simulink model "snap2_f_200msps_64i_4096c_for_8_11_2124fpg"  (R2019a)
settings: Solver=VariableStepDiscrete  StartTime=0.0  StopTime=10.0  RelTol=1e-3
undefined symbols (not defined in the file): datetime, posixtime
libraries: xbsIndex_r4 x17164, casper_library_misc x251, xps_library x14, casper_library_delays x12, casper_library_sources x4
(part 45 of 196)

== system snap2_f_200msps_64i_4096c_for_8_11_2124fpg/cosmic_ray/coincidence_trigger/bus_threshold 2 ==
  threshold : Inport
  bus_in : Inport  Port=2
  sync_in : Inport  Port=3
  Delay7 : xbsIndex_r4/Delay  rst=off  infoeditControl=Selection of Reset will increase slice count due to use of real FFs and instead of SRLs  en=off  latency=1  reg_retiming=off  dbl_ovrd=off  block_type=delay
  Relational{2..65} (64 alike) : xbsIndex_r4/Relational  mode=a>b  op_type=Bool  en=off  latency=1  dbl_ovrd=off  block_type=relational
  Scope{1..5,7} (6 alike) : Scope
  bus_create2 : Subsystem  AncestorBlock=casper_library_flow_control/bus_create  mask(bus_create){inputNum=64}
      mask prompts: inputNum "Number of inputs:"
      mask init:
      | bus_create_init(gcb,...
      |     'inputNum', inputNum);
  bus_expand : Subsystem  AncestorBlock=casper_library_flow_control/bus_expand  mask(bus_expand){mode=divisions of equal size, outputNum=64, outputWidth=20, outputBinaryPt=0, outputArithmeticType=0, show_format=off, outputToWorkspace=off, variablePrefix=out, outputToModelAsWell=on}
      mask prompts: outputNum "Number of outputs:"; outputWidth "Output width:"; outputBinaryPt "Output binary point position:"; outputArithmeticType "Output arithmetic type (ufix=0, fix=1, bool=2):"; show_format "Print format string?"; outputToWorkspace "Output to workspace?"; variablePrefix "Variable name prefix:"; outputToModelAsWell "Output to model as well?"
      mask help:
      | Expands a 'bus' made using a bus_create or Xilinx
      | concat block.
      | The input will be divided into the specified number
      | of outputs with the specified arithmetic types.
      | Divisions are made from most significant bit.
      mask init: same as snap2_f_200msps_64i_4096c_for_8_11_2124fpg/autocorr/bus_expand
      settings: AttributesFormatString=64 outputs
      mask TypeOptions mode: divisions of equal size | divisions of arbitrary size
      mask Callback mode: bus_expand_callback();
      mask Callback outputWidth: bus_expand_callback();
      mask Callback outputToWorkspace: bus_expand_callback();
  OUT : Outport
  sync_out : Outport  Port=2
  wires:
  bus_expand/24(out41) -> Relational33/1
  bus_expand/25(out40) -> Relational18/1
  bus_expand/14(out51) -> Relational15/1
  [thresh] -> Relational2/2
  bus_expand/42(out23) -> Relational35/1
  Relational6/1 -> bus_create2/5(in5)
  bus_expand/59(out6) -> Relational45/1
  bus_expand/54(out11) -> Relational57/1
  Relational57/1 -> bus_create2/54(in54)
  Relational34/1 -> bus_create2/41(in41)
  [thresh] -> Relational41/2
  Relational13/1 -> bus_create2/12(in12)
  Relational11/1 -> bus_create2/10(in10)
  [thresh] -> Relational65/2
  Relational2/1 -> bus_create2/1(in1), Scope2/1
  [thresh] -> Relational26/2
  bus_expand/6(out59) -> Relational7/1
  [thresh] -> Relational51/2
  bus_expand/47(out18) -> Relational40/1
  [thresh] -> Relational27/2
  [thresh] -> Relational47/2
  Relational24/1 -> bus_create2/31(in31)
  Relational62/1 -> bus_create2/37(in37)
  Relational10/1 -> bus_create2/9(in9)
  Relational48/1 -> bus_create2/62(in62)
  Relational18/1 -> bus_create2/25(in25)
  Relational23/1 -> bus_create2/30(in30)
  [thresh] -> Relational11/2
  Relational55/1 -> bus_create2/34(in34)
  Relational42/1 -> bus_create2/57(in57)
  Relational17/1 -> bus_create2/16(in16)
  Relational20/1 -> bus_create2/27(in27)
  Relational8/1 -> bus_create2/7(in7)
  bus_expand/55(out10) -> Relational58/1
  bus_expand/23(out42) -> Relational32/1
  bus_expand/27(out38) -> Relational20/1
  bus_expand/17(out48) -> Relational26/1
  Relational38/1 -> bus_create2/45(in45)
  [thresh] -> Relational37/2
  Relational41/1 -> bus_create2/48(in48)
  bus_expand/31(out34) -> Relational24/1
  [thresh] -> Relational46/2
  bus_in/1 -> bus_expand/1(bus_in)
  Relational30/1 -> bus_create2/21(in21)
  [thresh] -> Relational49/2
  bus_expand/39(out26) -> Relational64/1
  [thresh] -> Relational60/2
  [thresh] -> Relational17/2
  [thresh] -> Relational30/2
  Relational29/1 -> bus_create2/20(in20)
  Relational33/1 -> bus_create2/24(in24)
  Relational25/1 -> bus_create2/32(in32)
  bus_expand/43(out22) -> Relational36/1
  [thresh] -> Relational8/2
  Relational50/1 -> bus_create2/64(in64), Scope4/1
  bus_expand/13(out52) -> Relational14/1
  [thresh] -> Relational14/2
  bus_expand/4(out61) -> Relational5/1
  Relational43/1 -> bus_create2/58(in58)
  Relational44/1 -> bus_create2/33(in33)
  [thresh] -> Relational29/2
  Relational54/1 -> bus_create2/52(in52)
  bus_expand/48(out17) -> Relational41/1
  bus_expand/15(out50) -> Relational16/1
  Relational9/1 -> bus_create2/8(in8)
  bus_expand/20(out45) -> Relational29/1
  [thresh] -> Relational25/2
  bus_expand/3(out62) -> Relational4/1
  bus_expand/7(out58) -> Relational8/1
  [thresh] -> Relational59/2
  bus_expand/8(out57) -> Relational9/1
  [thresh] -> Relational16/2
  bus_expand/12(out53) -> Relational13/1
  [thresh] -> Relational62/2
  [thresh] -> Relational45/2
  Relational5/1 -> bus_create2/4(in4)
  bus_expand/52(out13) -> Relational54/1
  bus_expand/46(out19) -> Relational39/1
  bus_expand/36(out29) -> Relational61/1
  bus_expand/22(out43) -> Relational31/1
  [thresh] -> Relational64/2
  bus_expand/50(out15) -> Relational52/1
  [thresh] -> Relational15/2
  [thresh] -> Relational3/2
  Relational52/1 -> bus_create2/50(in50)
  [thresh] -> Relational38/2
  Relational32/1 -> bus_create2/23(in23)
  bus_expand/16(out49) -> Relational17/1
  [thresh] -> Relational12/2
  bus_expand/41(out24) -> Relational34/1
  bus_expand/28(out37) -> Relational21/1
  Relational12/1 -> bus_create2/11(in11)
  Relational19/1 -> bus_create2/26(in26)
  Relational40/1 -> bus_create2/47(in47)
  [thresh] -> Relational57/2
  Relational27/1 -> bus_create2/18(in18)
  [thresh] -> Relational42/2
  Relational49/1 -> bus_create2/63(in63)
  Relational60/1 -> bus_create2/35(in35)
  [thresh] -> Relational13/2
  Delay7/1 -> sync_out/1
  [thresh] -> Relational36/2
  bus_expand/62(out3) -> Relational48/1, Scope7/1
  bus_expand/33(out32) -> Relational44/1
  bus_create2/1(bus_out) -> OUT/1
  Relational28/1 -> bus_create2/19(in19)
  Relational31/1 -> bus_create2/22(in22)
  Relational65/1 -> bus_create2/40(in40)
  [thresh] -> Relational18/2
  [thresh] -> Relational54/2
  bus_expand/26(out39) -> Relational19/1
  [thresh] -> Relational55/2
  [thresh] -> Relational20/2
  [thresh] -> Relational22/2
  bus_expand/35(out30) -> Relational60/1
  Relational21/1 -> bus_create2/28(in28)
  Relational22/1 -> bus_create2/29(in29)
  bus_expand/18(out47) -> Relational27/1
  bus_expand/11(out54) -> Relational12/1
  [thresh] -> Relational39/2
  bus_expand/34(out31) -> Relational55/1
  [thresh] -> Relational31/2
  bus_expand/2(out63) -> Relational3/1
  [thresh] -> Relational9/2
  Relational26/1 -> bus_create2/17(in17)
  [thresh] -> Relational4/2
  Relational3/1 -> bus_create2/2(in2)
  [thresh] -> Relational24/2
  bus_expand/60(out5) -> Relational46/1
  bus_expand/32(out33) -> Relational25/1
  [thresh] -> Relational40/2
  Relational7/1 -> bus_create2/6(in6)
  bus_expand/21(out44) -> Relational30/1
  [thresh] -> Relational19/2
  Relational59/1 -> bus_create2/56(in56)
  [thresh] -> Relational32/2
  [thresh] -> Relational48/2
  [thresh] -> Relational21/2
  [thresh] -> Relational52/2
  bus_expand/10(out55) -> Relational11/1
  Relational39/1 -> bus_create2/46(in46)
  bus_expand/9(out56) -> Relational10/1
  [thresh] -> Relational10/2
  bus_expand/63(out2) -> Relational49/1, Scope5/1
  [thresh] -> Relational28/2
  Relational56/1 -> bus_create2/53(in53)
  bus_expand/57(out8) -> Relational42/1
  [thresh] -> Relational50/2
  Relational35/1 -> bus_create2/42(in42)
  bus_expand/38(out27) -> Relational63/1
  [thresh] -> Relational35/2
  bus_expand/1(msb_out64) -> Relational2/1, Scope1/1
  Relational58/1 -> bus_create2/55(in55)
  [thresh] -> Relational6/2
  bus_expand/64(lsb_out1) -> Relational50/1, Scope3/1
  Relational4/1 -> bus_create2/3(in3)
  [thresh] -> Relational53/2
  bus_expand/29(out36) -> Relational22/1
  [thresh] -> Relational34/2
  [thresh] -> Relational63/2
  [thresh] -> Relational58/2
  [thresh] -> Relational56/2
  Relational64/1 -> bus_create2/39(in39)
  bus_expand/56(out9) -> Relational59/1
  bus_expand/37(out28) -> Relational62/1
  [thresh] -> Relational43/2
  bus_expand/44(out21) -> Relational37/1
  Relational61/1 -> bus_create2/36(in36)
  Relational47/1 -> bus_create2/61(in61)
  Relational14/1 -> bus_create2/13(in13)
  bus_expand/19(out46) -> Relational28/1
  [thresh] -> Relational33/2
  bus_expand/5(out60) -> Relational6/1
  Relational51/1 -> bus_create2/49(in49)
  bus_expand/53(out12) -> Relational56/1
  sync_in/1 -> Delay7/1
  bus_expand/58(out7) -> Relational43/1
  Relational16/1 -> bus_create2/15(in15)
  bus_expand/51(out14) -> Relational53/1
  bus_expand/49(out16) -> Relational51/1
  bus_expand/30(out35) -> Relational23/1
  Relational53/1 -> bus_create2/51(in51)
  [thresh] -> Relational7/2
  Relational37/1 -> bus_create2/44(in44)
  threshold/1 -> [thresh]
  [thresh] -> Relational23/2
  Relational45/1 -> bus_create2/59(in59)
  [thresh] -> Relational44/2
  Relational63/1 -> bus_create2/38(in38)
  Relational36/1 -> bus_create2/43(in43)
  bus_expand/40(out25) -> Relational65/1
  Relational15/1 -> bus_create2/14(in14)
  [thresh] -> Relational5/2
  bus_expand/45(out20) -> Relational38/1
  bus_expand/61(out4) -> Relational47/1
  [thresh] -> Relational61/2
  Relational46/1 -> bus_create2/60(in60)

== system snap2_f_200msps_64i_4096c_for_8_11_2124fpg/cosmic_ray/coincidence_trigger/custom_pulse_extend_with_sync ==
  note: Subtract one so that the actual length of the extended pulse is the input number of clock cycles.
  "in " : Inport
  "pulse_length " : Inport  Port=2
  sync_in : Inport  Port=3
  AddSub : xbsIndex_r4/AddSub  mode=Subtraction  use_carryin=off  use_carryout=off  en=off  latency=1  precision=Full  arith_type=Unsigned  n_bits=16  bin_pt=14  quantization=Truncate  overflow=Wrap  use_behavioral_HDL=off  hw_selection=Fabric  pipelined=off  dbl_ovrd=off  block_type=addsub
  Constant22 : xbsIndex_r4/Constant  const=1  gui_display_data_type=Fixed-point  arith_type=Unsigned  n_bits=12  bin_pt=0  preci_type=Single  exp_width=8  frac_width=24  explicit_period=on  period=1  equ=P=C  opselect=C  inp2=PCIN>>17  opr=+  inp1=P  carry=CIN  dbl_ovrd=off  block_type=constant
  Counter : xbsIndex_r4/Counter  cnt_type=Free Running  cnt_to=Inf  operation=Up  start_count=0  cnt_by_val=1  arith_type=Unsigned  n_bits=12  bin_pt=0  load_pin=off  rst=on  en=on  explicit_period=on  period=1  use_behavioral_HDL=off  implementation=Fabric  dbl_ovrd=off  block_type=counter
  Delay1 : xbsIndex_r4/Delay  rst=off  infoeditControl=Selection of Reset will increase slice count due to use of real FFs and instead of SRLs  en=off  latency=2  reg_retiming=off  dbl_ovrd=off  block_type=delay
  Relational : xbsIndex_r4/Relational  mode=a<b  op_type=Bool  en=off  latency=1  dbl_ovrd=off  block_type=relational
  Scope1 : Scope
  edge_detect : casper_library_misc/edge_detect  edge=Rising  polarity=Active High  x_in=[0 0.08 0.08 0.16 0.16 0.24 0.24 0.32 0.32 0.4]  y_in="[-1 -1  1  1  1  1  1  1  1  1]"  x_out=[0.6 0.68 0.68 0.76 0.76 0.84 0.84 0.92 0.92 1]  y_out="[-1 -1  1  1 -1 -1 -1 -1 -1 -1]"
  "out " : Outport
  sync_out : Outport  Port=2
  wires:
  AddSub/1 -> Relational/2
  Relational/1 -> Counter/2, "out "/1
  sync_in/1 -> Delay1/1
  "pulse_length "/1 -> AddSub/1
  Constant22/1 -> AddSub/2
  "in "/1 -> Scope1/1, edge_detect/1
  edge_detect/1 -> Counter/1
  Delay1/1 -> sync_out/1
  Counter/1 -> Relational/1

== system snap2_f_200msps_64i_4096c_for_8_11_2124fpg/cosmic_ray/coincidence_trigger/custom_pulse_extend_with_sync1 ==
  note: Subtract one so that the actual length of the extended pulse is the input number of clock cycles.
  "in " : Inport
  "pulse_length " : Inport  Port=2
  sync_in : Inport  Port=3
  AddSub : xbsIndex_r4/AddSub  mode=Subtraction  use_carryin=off  use_carryout=off  en=off  latency=1  precision=Full  arith_type=Unsigned  n_bits=16  bin_pt=14  quantization=Truncate  overflow=Wrap  use_behavioral_HDL=off  hw_selection=Fabric  pipelined=off  dbl_ovrd=off  block_type=addsub
  Constant22 : xbsIndex_r4/Constant  const=1  gui_display_data_type=Fixed-point  arith_type=Unsigned  n_bits=12  bin_pt=0  preci_type=Single  exp_width=8  frac_width=24  explicit_period=on  period=1  equ=P=C  opselect=C  inp2=PCIN>>17  opr=+  inp1=P  carry=CIN  dbl_ovrd=off  block_type=constant
  Counter : xbsIndex_r4/Counter  cnt_type=Free Running  cnt_to=Inf  operation=Up  start_count=0  cnt_by_val=1  arith_type=Unsigned  n_bits=12  bin_pt=0  load_pin=off  rst=on  en=on  explicit_period=on  period=1  use_behavioral_HDL=off  implementation=Fabric  dbl_ovrd=off  block_type=counter
  Delay1 : xbsIndex_r4/Delay  rst=off  infoeditControl=Selection of Reset will increase slice count due to use of real FFs and instead of SRLs  en=off  latency=2  reg_retiming=off  dbl_ovrd=off  block_type=delay
  Relational : xbsIndex_r4/Relational  mode=a<b  op_type=Bool  en=off  latency=1  dbl_ovrd=off  block_type=relational
  Scope1 : Scope
  edge_detect : casper_library_misc/edge_detect  edge=Rising  polarity=Active High  x_in=[0 0.08 0.08 0.16 0.16 0.24 0.24 0.32 0.32 0.4]  y_in="[-1 -1  1  1  1  1  1  1  1  1]"  x_out=[0.6 0.68 0.68 0.76 0.76 0.84 0.84 0.92 0.92 1]  y_out="[-1 -1  1  1 -1 -1 -1 -1 -1 -1]"
  "out " : Outport
  sync_out : Outport  Port=2
  wires:
  Counter/1 -> Relational/1
  Delay1/1 -> sync_out/1
  edge_detect/1 -> Counter/1
  "in "/1 -> edge_detect/1, Scope1/1
  Constant22/1 -> AddSub/2
  "pulse_length "/1 -> AddSub/1
  sync_in/1 -> Delay1/1
  Relational/1 -> "out "/1, Counter/2
  AddSub/1 -> Relational/2

== system snap2_f_200msps_64i_4096c_for_8_11_2124fpg/cosmic_ray/coincidence_trigger/square_bus_components ==
  IN : Inport
  sync_in : Inport  Port=2
  Delay7 : xbsIndex_r4/Delay  rst=off  infoeditControl=Selection of Reset will increase slice count due to use of real FFs and instead of SRLs  en=off  latency=3  reg_retiming=off  dbl_ovrd=off  block_type=delay
  Mult{,1..63} (64 alike) : xbsIndex_r4/Mult  precision=Full  arith_type="Signed  (2's comp)"  n_bits=16  bin_pt=14  quantization=Truncate  overflow=Wrap  en=off  latency=3  use_behavioral_HDL=off  opt=Speed  use_embedded=on  optimum_pipeline=off  pipeline=on  dbl_ovrd=off  use_rpm=on  placement_style=Triangular  block_type=mult
  Scope{,1..5} (6 alike) : Scope
  bus_create2 : Subsystem  AncestorBlock=casper_library_flow_control/bus_create  mask(bus_create){inputNum=64}
      mask prompts: inputNum "Number of inputs:"
      mask init:
      | bus_create_init(gcb,...
      |     'inputNum', inputNum);
  bus_expand : Subsystem  AncestorBlock=casper_library_flow_control/bus_expand  mask(bus_expand){mode=divisions of equal size, outputNum=64, outputWidth=10, outputBinaryPt=0, outputArithmeticType=0, show_format=off, outputToWorkspace=off, variablePrefix=out, outputToModelAsWell=on}
      mask prompts: outputNum "Number of outputs:"; outputWidth "Output width:"; outputBinaryPt "Output binary point position:"; outputArithmeticType "Output arithmetic type (ufix=0, fix=1, bool=2):"; show_format "Print format string?"; outputToWorkspace "Output to workspace?"; variablePrefix "Variable name prefix:"; outputToModelAsWell "Output to model as well?"
      mask help:
      | Expands a 'bus' made using a bus_create or Xilinx
      | concat block.
      | The input will be divided into the specified number
      | of outputs with the specified arithmetic types.
      | Divisions are made from most significant bit.
      mask init: same as snap2_f_200msps_64i_4096c_for_8_11_2124fpg/autocorr/bus_expand
      settings: AttributesFormatString=64 outputs
      mask TypeOptions mode: divisions of equal size | divisions of arbitrary size
      mask Callback mode: bus_expand_callback();
      mask Callback outputWidth: bus_expand_callback();
      mask Callback outputToWorkspace: bus_expand_callback();
  OUT : Outport
  sync_out : Outport  Port=2
  wires:
  Mult38/1 -> bus_create2/47(in47)
  Mult31/1 -> bus_create2/26(in26)
  Mult35/1 -> bus_create2/44(in44)
  Mult36/1 -> bus_create2/45(in45)
  Mult4/1 -> bus_create2/5(in5)
  Mult22/1 -> bus_create2/31(in31)
  Mult5/1 -> bus_create2/6(in6)
  Mult59/1 -> bus_create2/38(in38)
  Mult20/1 -> bus_create2/29(in29)
  Mult16/1 -> bus_create2/17(in17)
  Mult43/1 -> bus_create2/60(in60)
  Mult45/1 -> Scope5/1, bus_create2/61(in61)
  Delay7/1 -> sync_out/1
  bus_expand/34(out31) -> Mult33/2, Mult33/1
  Mult47/1 -> bus_create2/63(in63), Scope3/1
  Mult46/1 -> Scope4/1, bus_create2/62(in62)
  bus_expand/44(out21) -> Mult35/2, Mult35/1
  bus_expand/7(out58) -> Mult6/1, Mult6/2
  Mult34/1 -> bus_create2/43(in43)
  bus_expand/33(out32) -> Mult32/2, Mult32/1
  bus_expand/29(out36) -> Mult20/2, Mult20/1
  bus_expand/49(out16) -> Mult40/1, Mult40/2
  bus_expand/60(out5) -> Mult43/1, Mult43/2
  Mult21/1 -> bus_create2/30(in30)
  bus_expand/3(out62) -> Mult2/2, Mult2/1
  Mult12/1 -> bus_create2/13(in13)
  bus_expand/26(out39) -> Mult31/1, Mult31/2
  bus_expand/9(out56) -> Mult8/2, Mult8/1
  bus_expand/19(out46) -> Mult24/1, Mult24/2
  sync_in/1 -> Delay7/1
  Mult14/1 -> bus_create2/15(in15)
  bus_expand/56(out9) -> Mult54/1, Mult54/2
  Mult15/1 -> bus_create2/16(in16)
  bus_expand/36(out29) -> Mult55/1, Mult55/2
  Mult39/1 -> bus_create2/48(in48)
  bus_expand/35(out30) -> Mult44/1, Mult44/2
  bus_expand/8(out57) -> Mult7/1, Mult7/2
  Mult37/1 -> bus_create2/46(in46)
  Mult42/1 -> bus_create2/59(in59)
  bus_expand/51(out14) -> Mult49/2, Mult49/1
  bus_expand/41(out24) -> Mult62/1, Mult62/2
  Mult24/1 -> bus_create2/19(in19)
  bus_expand/42(out23) -> Mult63/1, Mult63/2
  Mult9/1 -> bus_create2/10(in10)
  Mult30/1 -> bus_create2/25(in25)
  Mult32/1 -> bus_create2/33(in33)
  bus_expand/16(out49) -> Mult15/2, Mult15/1
  bus_expand/32(out33) -> Mult23/1, Mult23/2
  Mult7/1 -> bus_create2/8(in8)
  bus_expand/13(out52) -> Mult12/1, Mult12/2
  bus_expand/63(out2) -> Mult47/2, Mult47/1
  bus_expand/6(out59) -> Mult5/2, Mult5/1
  bus_expand/14(out51) -> Mult13/1, Mult13/2
  bus_expand/31(out34) -> Mult22/1, Mult22/2
  Mult54/1 -> bus_create2/56(in56)
  Mult23/1 -> bus_create2/32(in32)
  bus_expand/2(out63) -> Mult1/1, Mult1/2
  Mult58/1 -> bus_create2/37(in37)
  Mult28/1 -> bus_create2/23(in23)
  Mult51/1 -> bus_create2/53(in53)
  Mult10/1 -> bus_create2/11(in11)
  Mult26/1 -> bus_create2/21(in21)
  bus_expand/64(lsb_out1) -> Mult48/2, Mult48/1
  IN/1 -> bus_expand/1(bus_in)
  Mult48/1 -> bus_create2/64(in64), Scope2/1
  Mult17/1 -> bus_create2/18(in18)
  bus_expand/28(out37) -> Mult19/2, Mult19/1
  Mult29/1 -> bus_create2/24(in24)
  Mult8/1 -> bus_create2/9(in9)
  bus_expand/46(out19) -> Mult37/2, Mult37/1
  Mult11/1 -> bus_create2/12(in12)
  Mult50/1 -> bus_create2/52(in52)
  Mult41/1 -> bus_create2/50(in50)
  bus_expand/48(out17) -> Mult39/1, Mult39/2
  bus_expand/17(out48) -> Mult16/2, Mult16/1
  Mult63/1 -> bus_create2/42(in42)
  bus_expand/15(out50) -> Mult14/2, Mult14/1
  Mult57/1 -> bus_create2/58(in58)
  bus_expand/57(out8) -> Mult56/2, Mult56/1
  bus_expand/18(out47) -> Mult17/2, Mult17/1
  Mult60/1 -> bus_create2/39(in39)
  bus_expand/37(out28) -> Mult58/1, Mult58/2
  bus_expand/54(out11) -> Mult52/2, Mult52/1
  bus_expand/27(out38) -> Mult18/2, Mult18/1
  bus_expand/10(out55) -> Mult9/2, Mult9/1
  bus_expand/23(out42) -> Mult28/2, Mult28/1
  bus_expand/30(out35) -> Mult21/2, Mult21/1
  bus_expand/38(out27) -> Mult59/1, Mult59/2
  Mult13/1 -> bus_create2/14(in14)
  Mult18/1 -> bus_create2/27(in27)
  Mult49/1 -> bus_create2/51(in51)
  Mult3/1 -> bus_create2/4(in4)
  Mult2/1 -> bus_create2/3(in3)
  Mult53/1 -> bus_create2/55(in55)
  Mult40/1 -> bus_create2/49(in49)
  bus_expand/52(out13) -> Mult50/2, Mult50/1
  bus_expand/40(out25) -> Mult61/2, Mult61/1
  Mult52/1 -> bus_create2/54(in54)
  bus_expand/39(out26) -> Mult60/2, Mult60/1
  bus_expand/21(out44) -> Mult26/1, Mult26/2
  bus_expand/5(out60) -> Mult4/2, Mult4/1
  bus_expand/50(out15) -> Mult41/1, Mult41/2
  bus_expand/45(out20) -> Mult36/2, Mult36/1
  bus_expand/61(out4) -> Mult45/1, Mult45/2
  Mult27/1 -> bus_create2/22(in22)
  Mult25/1 -> bus_create2/20(in20)
  Mult19/1 -> bus_create2/28(in28)
  bus_create2/1(bus_out) -> OUT/1
  Mult1/1 -> Scope1/1, bus_create2/2(in2)
  bus_expand/47(out18) -> Mult38/1, Mult38/2
  bus_expand/59(out6) -> Mult42/1, Mult42/2
  bus_expand/1(msb_out64) -> Mult/1, Mult/2
  bus_expand/43(out22) -> Mult34/2, Mult34/1
  bus_expand/25(out40) -> Mult30/1, Mult30/2
  Mult6/1 -> bus_create2/7(in7)
  bus_expand/55(out10) -> Mult53/1, Mult53/2
  bus_expand/58(out7) -> Mult57/2, Mult57/1
  bus_expand/53(out12) -> Mult51/2, Mult51/1
  Mult33/1 -> bus_create2/34(in34)
  bus_expand/24(out41) -> Mult29/2, Mult29/1
  Mult62/1 -> bus_create2/41(in41)
  bus_expand/11(out54) -> Mult10/1, Mult10/2
  bus_expand/4(out61) -> Mult3/2, Mult3/1
  Mult61/1 -> bus_create2/40(in40)
  bus_expand/12(out53) -> Mult11/1, Mult11/2
  Mult55/1 -> bus_create2/36(in36)
  Mult44/1 -> bus_create2/35(in35)
  Mult/1 -> Scope/1, bus_create2/1(in1)
  Mult56/1 -> bus_create2/57(in57)
  bus_expand/62(out3) -> Mult46/1, Mult46/2
  bus_expand/22(out43) -> Mult27/1, Mult27/2
  bus_expand/20(out45) -> Mult25/1, Mult25/2

== system snap2_f_200msps_64i_4096c_for_8_11_2124fpg/cosmic_ray/coincidence_trigger/trigger_coincidencer ==
  window_length : Inport
  data_bus_in : Inport  Port=2
  sync_in : Inport  Port=3
  Scope : Scope
  Scope1 : Scope
  adder_tree : Subsystem  AncestorBlock=casper_library_misc/adder_tree  mask(adder_tree){n_inputs=64, csp_latency=1, adder_imp=Fabric, first_stage_hdl=on, floating_point=off, dvalid_en=off, float_type=single, frac_width=0, exp_width=0}
      mask prompts: n_inputs "Number of Inputs"; csp_latency "Add Latency"; adder_imp "Adder implementation"; first_stage_hdl "Use behavioural HDL for first stage of adders"; floating_point "floating point"; float_type "float type"; frac_width "fraction length"; exp_width "exponent length"
      mask help:
      | Sums all inputs using a tree of adds and delays.
      |
      | Number of inputs: The number of inputs to the block to be summed
      | Add latency: The number of clock cycles to perform one addition (affects timing)..
      | Adder implementation: choice of fabric or DSP48E core, or behavioral HDL
      | Use behavioural HDL for first stage of adders: Causes the first stage of adders to be absorbed into DSP blocks if multipliers precede them (Virtex 5). Overrides Adder implementation
      mask init:
      | adder_tree_init(gcb, ...
      |     'n_inputs', n_inputs, ...
      |     'csp_latency', csp_latency, ...
      |     'adder_imp', adder_imp, ...
      |     'dvalid_en', dvalid_en, ...
      |     'floating_point', floating_point, ...
      |     'float_type', float_type, ...
      |     'exp_width', exp_width, ...
      |     'frac_width', frac_width, ...     
      |     'first_stage_hdl', first_stage_hdl);
      settings: AttributesFormatString=latency 6
      mask Help: eval('xlWeb(''http://casper.berkeley.edu/wiki/Adder_tree'')')
      mask TypeOptions adder_imp: Behavioral | Fabric | DSP48
      mask Callback floating_point:
      | onoff = get_param(gcb, 'floating_point');
      | if strcmp(onoff,'on')
      |     enable_fixpt = 'off'
      |     set_param_state(gcb, 'float_type', 'on')
      | 
      |     f_type = get_param(gcb, 'float_type')
      |     if strcmp(f_type,'custom')
      |         set_param_state(gcb, 'exp_width', 'on')
      |         set_param_state(gcb, 'frac_width', 'on')
      |     else
      |         set_param_state(gcb, 'exp_width', 'off')
      |         set_param_state(gcb, 'frac_width', 'off')
      |     end
      | 
      | 
      | else
      |     enable_fixpt = 'on'
      |     set_param_state(gcb, 'float_type', 'off')
      |     set_param_state(gcb, 'exp_width', 'off')
      |     set_param_state(gcb, 'frac_width', 'off')
      | end
      mask TypeOptions float_type: single | custom
      mask Callback float_type:
      | f_type = get_param(gcb, 'float_type')
      | if strcmp(f_type,'custom')
      |     set_param_state(gcb, 'exp_width', 'on')
      |     set_param_state(gcb, 'frac_width', 'on')
      | else
      |     set_param_state(gcb, 'exp_width', 'off')
      |     set_param_state(gcb, 'frac_width', 'off')
      | end
  bus_expand : Subsystem  AncestorBlock=casper_library_flow_control/bus_expand  mask(bus_expand){mode=divisions of equal size, outputNum=64, outputWidth=1, outputBinaryPt=0, outputArithmeticType=0, show_format=off, outputToWorkspace=off, variablePrefix=out, outputToModelAsWell=on}
      mask prompts: outputNum "Number of outputs:"; outputWidth "Output width:"; outputBinaryPt "Output binary point position:"; outputArithmeticType "Output arithmetic type (ufix=0, fix=1, bool=2):"; show_format "Print format string?"; outputToWorkspace "Output to workspace?"; variablePrefix "Variable name prefix:"; outputToModelAsWell "Output to model as well?"
      mask help:
      | Expands a 'bus' made using a bus_create or Xilinx
      | concat block.
      | The input will be divided into the specified number
      | of outputs with the specified arithmetic types.
      | Divisions are made from most significant bit.
      mask init: same as snap2_f_200msps_64i_4096c_for_8_11_2124fpg/autocorr/bus_expand
      settings: AttributesFormatString=64 outputs
      mask TypeOptions mode: divisions of equal size | divisions of arbitrary size
      mask Callback mode: bus_expand_callback();
      mask Callback outputWidth: bus_expand_callback();
      mask Callback outputToWorkspace: bus_expand_callback();
  custom_pulse_extend1 : Subsystem
  custom_pulse_extend10 : Subsystem
  custom_pulse_extend11 : Subsystem
  custom_pulse_extend12 : Subsystem
  custom_pulse_extend13 : Subsystem
  custom_pulse_extend14 : Subsystem
  custom_pulse_extend15 : Subsystem
  custom_pulse_extend16 : Subsystem
  custom_pulse_extend17 : Subsystem
  custom_pulse_extend18 : Subsystem
  custom_pulse_extend19 : Subsystem
  custom_pulse_extend2 : Subsystem
  custom_pulse_extend20 : Subsystem
  custom_pulse_extend21 : Subsystem
  custom_pulse_extend22 : Subsystem
  custom_pulse_extend23 : Subsystem
  custom_pulse_extend24 : Subsystem
  custom_pulse_extend25 : Subsystem
  custom_pulse_extend26 : Subsystem
  custom_pulse_extend27 : Subsystem
  custom_pulse_extend28 : Subsystem
  custom_pulse_extend29 : Subsystem
  custom_pulse_extend3 : Subsystem
  custom_pulse_extend30 : Subsystem
  custom_pulse_extend31 : Subsystem
  custom_pulse_extend32 : Subsystem
  custom_pulse_extend33 : Subsystem
  custom_pulse_extend34 : Subsystem
  custom_pulse_extend35 : Subsystem
  custom_pulse_extend36 : Subsystem
  custom_pulse_extend37 : Subsystem
  custom_pulse_extend38 : Subsystem
  custom_pulse_extend39 : Subsystem
  custom_pulse_extend4 : Subsystem
  custom_pulse_extend40 : Subsystem
  custom_pulse_extend41 : Subsystem
  custom_pulse_extend42 : Subsystem
  custom_pulse_extend43 : Subsystem
  custom_pulse_extend44 : Subsystem
  custom_pulse_extend46 : Subsystem
  custom_pulse_extend47 : Subsystem
  custom_pulse_extend48 : Subsystem
  custom_pulse_extend49 : Subsystem
  custom_pulse_extend5 : Subsystem
  custom_pulse_extend50 : Subsystem
  custom_pulse_extend51 : Subsystem
  custom_pulse_extend52 : Subsystem
  custom_pulse_extend53 : Subsystem
  custom_pulse_extend54 : Subsystem
  custom_pulse_extend55 : Subsystem
  custom_pulse_extend56 : Subsystem
  custom_pulse_extend57 : Subsystem
  custom_pulse_extend58 : Subsystem
  custom_pulse_extend59 : Subsystem
  custom_pulse_extend6 : Subsystem
  custom_pulse_extend60 : Subsystem
  custom_pulse_extend61 : Subsystem
  custom_pulse_extend62 : Subsystem
  custom_pulse_extend63 : Subsystem
  custom_pulse_extend64 : Subsystem
  custom_pulse_extend7 : Subsystem
  custom_pulse_extend8 : Subsystem
  custom_pulse_extend9 : Subsystem
  custom_pulse_extend_with_sync2 : Subsystem
  sync_out : Outport
  "out " : Outport  Port=2
  wires:
  bus_expand/24(out41) -> custom_pulse_extend35/1("in ")
  bus_expand/46(out19) -> custom_pulse_extend24/1("in ")
  custom_pulse_extend2/1("out ") -> adder_tree/62(din61)
  bus_expand/16(out49) -> custom_pulse_extend51/1("in ")
  custom_pulse_extend17/1("out ") -> adder_tree/39(din38)
  bus_expand/12(out53) -> custom_pulse_extend54/1("in ")
  bus_expand/7(out58) -> custom_pulse_extend56/1("in ")
  custom_pulse_extend21/1("out ") -> adder_tree/35(din34)
  bus_expand/23(out42) -> custom_pulse_extend63/1("in ")
  bus_expand/63(out2) -> custom_pulse_extend1/1("in ")
  bus_expand/1(msb_out64) -> custom_pulse_extend_with_sync2/1("in ")
  custom_pulse_extend42/1("out ") -> adder_tree/9(din8)
  bus_expand/2(out63) -> custom_pulse_extend46/1("in ")
  bus_expand/39(out26) -> custom_pulse_extend30/1("in ")
  custom_pulse_extend57/1("out ") -> adder_tree/6(din5)
  bus_expand/17(out48) -> custom_pulse_extend36/1("in ")
  bus_expand/42(out23) -> custom_pulse_extend28/1("in ")
  bus_expand/18(out47) -> custom_pulse_extend37/1("in ")
  bus_expand/19(out46) -> custom_pulse_extend34/1("in ")
  bus_expand/20(out45) -> custom_pulse_extend38/1("in ")
  custom_pulse_extend27/1("out ") -> adder_tree/42(din41)
  custom_pulse_extend18/1("out ") -> adder_tree/36(din35)
  custom_pulse_extend4/1("out ") -> adder_tree/60(din59)
  custom_pulse_extend53/1("out ") -> adder_tree/11(din10)
  bus_expand/49(out16) -> custom_pulse_extend13/1("in ")
  bus_expand/11(out54) -> custom_pulse_extend50/1("in ")
  custom_pulse_extend15/1("out ") -> adder_tree/53(din52)
  bus_expand/14(out51) -> custom_pulse_extend49/1("in ")
  custom_pulse_extend37/1("out ") -> adder_tree/19(din18)
  bus_expand/54(out11) -> custom_pulse_extend10/1("in ")
  bus_expand/25(out40) -> custom_pulse_extend60/1("in ")
  bus_expand/33(out32) -> custom_pulse_extend20/1("in ")
  bus_expand/53(out12) -> custom_pulse_extend9/1("in ")
  bus_expand/37(out28) -> custom_pulse_extend31/1("in ")
  bus_expand/22(out43) -> custom_pulse_extend33/1("in ")
  custom_pulse_extend32/1("out ") -> adder_tree/32(din31)
  bus_expand/51(out14) -> custom_pulse_extend11/1("in ")
  custom_pulse_extend5/1("out ") -> adder_tree/58(din57)
  custom_pulse_extend16/1("out ") -> adder_tree/48(din47)
  bus_expand/31(out34) -> custom_pulse_extend32/1("in ")
  custom_pulse_extend47/1("out ") -> adder_tree/5(din4)
  bus_expand/21(out44) -> custom_pulse_extend64/1("in ")
  bus_expand/57(out8) -> custom_pulse_extend5/1("in ")
  custom_pulse_extend33/1("out ") -> adder_tree/23(din22)
  custom_pulse_extend35/1("out ") -> adder_tree/25(din24)
  custom_pulse_extend36/1("out ") -> adder_tree/18(din17)
  custom_pulse_extend63/1("out ") -> adder_tree/24(din23)
  bus_expand/27(out38) -> custom_pulse_extend58/1("in ")
  custom_pulse_extend22/1("out ") -> adder_tree/37(din36)
  bus_expand/43(out22) -> custom_pulse_extend25/1("in ")
  custom_pulse_extend31/1("out ") -> adder_tree/38(din37)
  bus_expand/58(out7) -> custom_pulse_extend6/1("in ")
  custom_pulse_extend52/1("out ") -> adder_tree/10(din9)
  bus_expand/35(out30) -> custom_pulse_extend18/1("in ")
  bus_expand/36(out29) -> custom_pulse_extend22/1("in ")
  custom_pulse_extend60/1("out ") -> adder_tree/26(din25)
  bus_expand/60(out5) -> custom_pulse_extend7/1("in ")
  custom_pulse_extend64/1("out ") -> adder_tree/22(din21)
  bus_expand/13(out52) -> custom_pulse_extend48/1("in ")
  bus_expand/40(out25) -> custom_pulse_extend19/1("in ")
  bus_expand/52(out13) -> custom_pulse_extend15/1("in ")
  bus_expand/15(out50) -> custom_pulse_extend39/1("in ")
  custom_pulse_extend58/1("out ") -> adder_tree/28(din27)
  bus_expand/59(out6) -> custom_pulse_extend4/1("in ")
  bus_expand/29(out36) -> custom_pulse_extend43/1("in ")
  custom_pulse_extend59/1("out ") -> adder_tree/33(din32)
  window_length/1 -> custom_pulse_extend46/2("pulse_length "), custom_pulse_extend41/2("pulse_length "), custom_pulse_extend47/2("pulse_length "), custom_pulse_extend57/2("pulse_length "), custom_pulse_extend40/2("pulse_length "), custom_pulse_extend56/2("pulse_length "), custom_pulse_extend42/2("pulse_length "), custom_pulse_extend52/2("pulse_length "), custom_pulse_extend53/2("pulse_length "), custom_pulse_extend50/2("pulse_length "), custom_pulse_extend54/2("pulse_length "), custom_pulse_extend48/2("pulse_length "), custom_pulse_extend49/2("pulse_length "), custom_pulse_extend39/2("pulse_length "), custom_pulse_extend51/2("pulse_length "), custom_pulse_extend36/2("pulse_length "), custom_pulse_extend37/2("pulse_length "), custom_pulse_extend34/2("pulse_length "), custom_pulse_extend38/2("pulse_length "), custom_pulse_extend33/2("pulse_length "), custom_pulse_extend35/2("pulse_length "), custom_pulse_extend60/2("pulse_length "), custom_pulse_extend61/2("pulse_length "), custom_pulse_extend58/2("pulse_length "), custom_pulse_extend62/2("pulse_length "), custom_pulse_extend43/2("pulse_length "), custom_pulse_extend55/2("pulse_length "), custom_pulse_extend32/2("pulse_length "), custom_pulse_extend59/2("pulse_length "), custom_pulse_extend20/2("pulse_length "), custom_pulse_extend21/2("pulse_length "), custom_pulse_extend18/2("pulse_length "), custom_pulse_extend22/2("pulse_length "), custom_pulse_extend31/2("pulse_length "), custom_pulse_extend17/2("pulse_length "), custom_pulse_extend30/2("pulse_length "), custom_pulse_extend19/2("pulse_length "), custom_pulse_extend27/2("pulse_length "), custom_pulse_extend28/2("pulse_length "), custom_pulse_extend25/2("pulse_length "), custom_pulse_extend29/2("pulse_length "), custom_pulse_extend23/2("pulse_length "), custom_pulse_extend24/2("pulse_length "), custom_pulse_extend16/2("pulse_length "), custom_pulse_extend26/2("pulse_length "), custom_pulse_extend13/2("pulse_length "), custom_pulse_extend14/2("pulse_length "), custom_pulse_extend11/2("pulse_length "), custom_pulse_extend15/2("pulse_length "), custom_pulse_extend9/2("pulse_length "), custom_pulse_extend10/2("pulse_length "), custom_pulse_extend8/2("pulse_length "), custom_pulse_extend12/2("pulse_length "), custom_pulse_extend5/2("pulse_length "), custom_pulse_extend6/2("pulse_length "), custom_pulse_extend4/2("pulse_length "), custom_pulse_extend7/2("pulse_length "), custom_pulse_extend2/2("pulse_length "), custom_pulse_extend3/2("pulse_length "), custom_pulse_extend1/2("pulse_length "), custom_pulse_extend44/2("pulse_length "), custom_pulse_extend63/2("pulse_length "), custom_pulse_extend64/2("pulse_length "), custom_pulse_extend_with_sync2/2("pulse_length ")
  custom_pulse_extend_with_sync2/1("out ") -> Scope1/1, adder_tree/2(din1)
  custom_pulse_extend40/1("out ") -> adder_tree/7(din6)
  custom_pulse_extend38/1("out ") -> adder_tree/21(din20)
  bus_expand/44(out21) -> custom_pulse_extend29/1("in ")
  custom_pulse_extend1/1("out ") -> adder_tree/64(din63)
  bus_expand/56(out9) -> custom_pulse_extend12/1("in ")
  custom_pulse_extend56/1("out ") -> adder_tree/8(din7)
  bus_expand/62(out3) -> custom_pulse_extend3/1("in ")
  bus_expand/10(out55) -> custom_pulse_extend53/1("in ")
  custom_pulse_extend34/1("out ") -> adder_tree/20(din19)
  custom_pulse_extend50/1("out ") -> adder_tree/12(din11)
  custom_pulse_extend10/1("out ") -> adder_tree/55(din54)
  data_bus_in/1 -> bus_expand/1(bus_in)
  custom_pulse_extend13/1("out ") -> adder_tree/50(din49)
  custom_pulse_extend26/1("out ") -> adder_tree/49(din48)
  bus_expand/6(out59) -> custom_pulse_extend40/1("in ")
  bus_expand/28(out37) -> custom_pulse_extend62/1("in ")
  adder_tree/2(dout) -> "out "/1
  sync_in/1 -> custom_pulse_extend_with_sync2/3(sync_in)
  custom_pulse_extend7/1("out ") -> adder_tree/61(din60)
  bus_expand/32(out33) -> custom_pulse_extend59/1("in ")
  bus_expand/47(out18) -> custom_pulse_extend16/1("in ")
  bus_expand/48(out17) -> custom_pulse_extend26/1("in ")
  custom_pulse_extend28/1("out ") -> adder_tree/43(din42)
  custom_pulse_extend6/1("out ") -> adder_tree/59(din58)
  custom_pulse_extend30/1("out ") -> adder_tree/40(din39)
  custom_pulse_extend14/1("out ") -> adder_tree/51(din50)
  bus_expand/64(lsb_out1) -> custom_pulse_extend44/1("in ")
  custom_pulse_extend43/1("out ") -> adder_tree/30(din29)
  bus_expand/34(out31) -> custom_pulse_extend21/1("in ")
  bus_expand/9(out56) -> custom_pulse_extend52/1("in ")
  custom_pulse_extend62/1("out ") -> adder_tree/29(din28)
  bus_expand/30(out35) -> custom_pulse_extend55/1("in ")
  bus_expand/5(out60) -> custom_pulse_extend57/1("in ")
  bus_expand/55(out10) -> custom_pulse_extend8/1("in ")
  custom_pulse_extend24/1("out ") -> adder_tree/47(din46)
  custom_pulse_extend48/1("out ") -> adder_tree/14(din13)
  custom_pulse_extend54/1("out ") -> adder_tree/13(din12)
  bus_expand/8(out57) -> custom_pulse_extend42/1("in ")
  custom_pulse_extend20/1("out ") -> adder_tree/34(din33)
  bus_expand/41(out24) -> custom_pulse_extend27/1("in ")
  bus_expand/61(out4) -> custom_pulse_extend2/1("in ")
  custom_pulse_extend61/1("out ") -> adder_tree/27(din26)
  bus_expand/4(out61) -> custom_pulse_extend47/1("in ")
  bus_expand/38(out27) -> custom_pulse_extend17/1("in ")
  bus_expand/45(out20) -> custom_pulse_extend23/1("in ")
  custom_pulse_extend9/1("out ") -> adder_tree/54(din53)
  custom_pulse_extend39/1("out ") -> adder_tree/16(din15)
  bus_expand/3(out62) -> custom_pulse_extend41/1("in ")
  custom_pulse_extend8/1("out ") -> adder_tree/56(din55)
  bus_expand/26(out39) -> custom_pulse_extend61/1("in ")
  adder_tree/1(sync_out) -> sync_out/1
  custom_pulse_extend55/1("out ") -> adder_tree/31(din30)
  bus_expand/50(out15) -> custom_pulse_extend14/1("in ")
  custom_pulse_extend23/1("out ") -> adder_tree/46(din45)
  custom_pulse_extend19/1("out ") -> adder_tree/41(din40)
  custom_pulse_extend51/1("out ") -> adder_tree/17(din16)
  custom_pulse_extend12/1("out ") -> adder_tree/57(din56)
  custom_pulse_extend49/1("out ") -> adder_tree/15(din14)
  custom_pulse_extend25/1("out ") -> adder_tree/44(din43)
  custom_pulse_extend29/1("out ") -> adder_tree/45(din44)
  custom_pulse_extend41/1("out ") -> adder_tree/4(din3)
  custom_pulse_extend46/1("out ") -> adder_tree/3(din2)
  custom_pulse_extend11/1("out ") -> adder_tree/52(din51)
  custom_pulse_extend3/1("out ") -> adder_tree/63(din62)
  custom_pulse_extend_with_sync2/2(sync_out) -> adder_tree/1(sync)
  custom_pulse_extend44/1("out ") -> Scope/1, adder_tree/65(din64)
